simulink model "vegas01_4tap_1024ch_220_r8a"  (Simulink 7.6)
settings: Solver=ode45  StartTime=0.0  StopTime=128000  RelTol=1e-3
libraries: xbsIndex_r4 x13459, monroe_library x80, xps_library x51, casper_library_misc x43, xbsReplacements_r4 x42, dspsigops x16, simulink x7
(part 4 of 99)

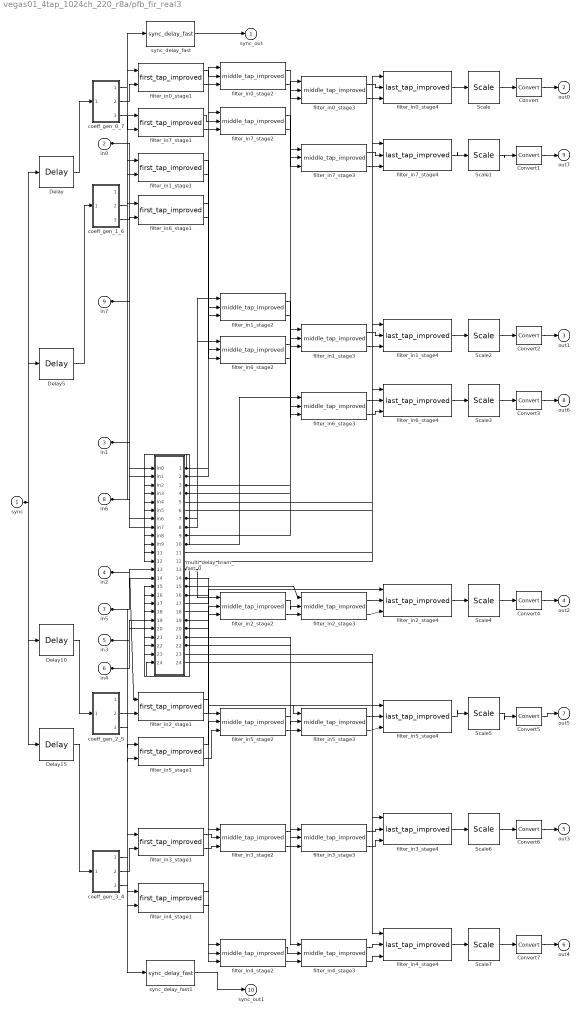
== system vegas01_4tap_1024ch_220_r8a/pfb_fir_real3 ==
  sync : Inport
  in0 : Inport  Port=2
  in1 : Inport  Port=3
  in2 : Inport  Port=4
  in3 : Inport  Port=5
  in4 : Inport  Port=6
  in5 : Inport  Port=7
  in6 : Inport  Port=8
  in7 : Inport  Port=9
  Convert{,1..7} (8 alike) : xbsIndex_r4/Convert  gui_display_data_type=Fixed-point  arith_type="Signed  (2's comp)"  n_bits=18  bin_pt=17  float_type=Single  exp_bits=8  fraction_bits=24  quantization=Truncate  overflow=Wrap  en=off  latency=0  dbl_ovrd=off  pipeline=off  block_type=convert
  Delay{,5,10,15} (4 alike) : xbsIndex_r4/Delay  rst=off  infoeditControl=Selection of Reset will increase slice count due to use of real FFs and instead of SRLs  en=off  latency=6  dbl_ovrd=off  reg_retiming=off  block_type=delay
  Scale{,1..7} (8 alike) : xbsIndex_r4/Scale  scale_factor=-1  dbl_ovrd=off  block_type=scale
  coeff_gen_0_7 : Subsystem
  coeff_gen_1_6 : Subsystem
  coeff_gen_2_5 : Subsystem
  coeff_gen_3_4 : Subsystem
  filter_in0_stage1 : monroe_library/first_tap_improved  SystemSampleTime=-1  data_bin_pt=7  coeff_bit_width=18
  filter_in0_stage2 : monroe_library/middle_tap_improved  SystemSampleTime=-1  data_bin_pt=7  coeff_bit_width=18  stage_num=2
  filter_in0_stage3 : monroe_library/middle_tap_improved  SystemSampleTime=-1  data_bin_pt=7  coeff_bit_width=18  stage_num=3
  filter_in0_stage4 : monroe_library/last_tap_improved  SystemSampleTime=-1  data_bin_pt=7  coeff_bin_pt=17  stage_num=4
  filter_in1_stage1 : monroe_library/first_tap_improved  SystemSampleTime=-1  data_bin_pt=7  coeff_bit_width=18
  filter_in1_stage2 : monroe_library/middle_tap_improved  SystemSampleTime=-1  data_bin_pt=7  coeff_bit_width=18  stage_num=2
  filter_in1_stage3 : monroe_library/middle_tap_improved  SystemSampleTime=-1  data_bin_pt=7  coeff_bit_width=18  stage_num=3
  filter_in1_stage4 : monroe_library/last_tap_improved  SystemSampleTime=-1  data_bin_pt=7  coeff_bin_pt=17  stage_num=4
  filter_in2_stage1 : monroe_library/first_tap_improved  SystemSampleTime=-1  data_bin_pt=7  coeff_bit_width=18
  filter_in2_stage2 : monroe_library/middle_tap_improved  SystemSampleTime=-1  data_bin_pt=7  coeff_bit_width=18  stage_num=2
  filter_in2_stage3 : monroe_library/middle_tap_improved  SystemSampleTime=-1  data_bin_pt=7  coeff_bit_width=18  stage_num=3
  filter_in2_stage4 : monroe_library/last_tap_improved  SystemSampleTime=-1  data_bin_pt=7  coeff_bin_pt=17  stage_num=4
  filter_in3_stage1 : monroe_library/first_tap_improved  SystemSampleTime=-1  data_bin_pt=7  coeff_bit_width=18
  filter_in3_stage2 : monroe_library/middle_tap_improved  SystemSampleTime=-1  data_bin_pt=7  coeff_bit_width=18  stage_num=2
  filter_in3_stage3 : monroe_library/middle_tap_improved  SystemSampleTime=-1  data_bin_pt=7  coeff_bit_width=18  stage_num=3
  filter_in3_stage4 : monroe_library/last_tap_improved  SystemSampleTime=-1  data_bin_pt=7  coeff_bin_pt=17  stage_num=4
  filter_in4_stage1 : monroe_library/first_tap_improved  SystemSampleTime=-1  data_bin_pt=7  coeff_bit_width=18
  filter_in4_stage2 : monroe_library/middle_tap_improved  SystemSampleTime=-1  data_bin_pt=7  coeff_bit_width=18  stage_num=2
  filter_in4_stage3 : monroe_library/middle_tap_improved  SystemSampleTime=-1  data_bin_pt=7  coeff_bit_width=18  stage_num=3
  filter_in4_stage4 : monroe_library/last_tap_improved  SystemSampleTime=-1  data_bin_pt=7  coeff_bin_pt=17  stage_num=4
  filter_in5_stage1 : monroe_library/first_tap_improved  SystemSampleTime=-1  data_bin_pt=7  coeff_bit_width=18
  filter_in5_stage2 : monroe_library/middle_tap_improved  SystemSampleTime=-1  data_bin_pt=7  coeff_bit_width=18  stage_num=2
  filter_in5_stage3 : monroe_library/middle_tap_improved  SystemSampleTime=-1  data_bin_pt=7  coeff_bit_width=18  stage_num=3
  filter_in5_stage4 : monroe_library/last_tap_improved  SystemSampleTime=-1  data_bin_pt=7  coeff_bin_pt=17  stage_num=4
  filter_in6_stage1 : monroe_library/first_tap_improved  SystemSampleTime=-1  data_bin_pt=7  coeff_bit_width=18
  filter_in6_stage2 : monroe_library/middle_tap_improved  SystemSampleTime=-1  data_bin_pt=7  coeff_bit_width=18  stage_num=2
  filter_in6_stage3 : monroe_library/middle_tap_improved  SystemSampleTime=-1  data_bin_pt=7  coeff_bit_width=18  stage_num=3
  filter_in6_stage4 : monroe_library/last_tap_improved  SystemSampleTime=-1  data_bin_pt=7  coeff_bin_pt=17  stage_num=4
  filter_in7_stage1 : monroe_library/first_tap_improved  SystemSampleTime=-1  data_bin_pt=7  coeff_bit_width=18
  filter_in7_stage2 : monroe_library/middle_tap_improved  SystemSampleTime=-1  data_bin_pt=7  coeff_bit_width=18  stage_num=2
  filter_in7_stage3 : monroe_library/middle_tap_improved  SystemSampleTime=-1  data_bin_pt=7  coeff_bit_width=18  stage_num=3
  filter_in7_stage4 : monroe_library/last_tap_improved  SystemSampleTime=-1  data_bin_pt=7  coeff_bin_pt=17  stage_num=4
  multi_delay_bram_fast_0 : Subsystem
  sync_delay_fast : monroe_library/sync_delay_fast  SystemSampleTime=-1  delay_len=768
      link override Constant2: opselect=C  inp2=PCIN>>17  inp1=P  carry=CIN
  sync_delay_fast1 : monroe_library/sync_delay_fast  SystemSampleTime=-1  delay_len=768
      link override Constant2: opselect=C  inp2=PCIN>>17  inp1=P  carry=CIN
  sync_out : Outport
  out0 : Outport  Port=2
  out1 : Outport  Port=3
  out2 : Outport  Port=4
  out3 : Outport  Port=5
  out4 : Outport  Port=6
  out5 : Outport  Port=7
  out6 : Outport  Port=8
  out7 : Outport  Port=9
  sync_out1 : Outport  Port=10
  wires:
  sync/1 -> Delay15/1, Delay10/1, Delay5/1, Delay/1
  in0/1 -> multi_delay_bram_fast_0/1(in0), filter_in0_stage1/1
  in7/1 -> multi_delay_bram_fast_0/2(in1), filter_in7_stage1/1
  in1/1 -> filter_in1_stage1/1, multi_delay_bram_fast_0/7(in6)
  in6/1 -> filter_in6_stage1/1, multi_delay_bram_fast_0/8(in7)
  in2/1 -> filter_in2_stage1/1, multi_delay_bram_fast_0/13(in12)
  in5/1 -> filter_in5_stage1/1, multi_delay_bram_fast_0/14(in13)
  in3/1 -> filter_in3_stage1/1, multi_delay_bram_fast_0/19(in18)
  in4/1 -> filter_in4_stage1/1, multi_delay_bram_fast_0/20(in19)
  multi_delay_bram_fast_0/1(out0) -> filter_in0_stage2/1, multi_delay_bram_fast_0/3(in2)
  multi_delay_bram_fast_0/2(out1) -> filter_in7_stage2/1, multi_delay_bram_fast_0/4(in3)
  multi_delay_bram_fast_0/3(out2) -> filter_in0_stage3/1, multi_delay_bram_fast_0/5(in4)
  multi_delay_bram_fast_0/4(out3) -> filter_in7_stage3/1, multi_delay_bram_fast_0/6(in5)
  multi_delay_bram_fast_0/7(out6) -> filter_in1_stage2/1, multi_delay_bram_fast_0/9(in8)
  multi_delay_bram_fast_0/8(out7) -> filter_in6_stage2/1, multi_delay_bram_fast_0/10(in9)
  multi_delay_bram_fast_0/9(out8) -> filter_in1_stage3/1, multi_delay_bram_fast_0/11(in10)
  multi_delay_bram_fast_0/10(out9) -> filter_in6_stage3/1, multi_delay_bram_fast_0/12(in11)
  multi_delay_bram_fast_0/13(out12) -> filter_in2_stage2/1, multi_delay_bram_fast_0/15(in14)
  multi_delay_bram_fast_0/14(out13) -> filter_in5_stage2/1, multi_delay_bram_fast_0/16(in15)
  multi_delay_bram_fast_0/15(out14) -> filter_in2_stage3/1, multi_delay_bram_fast_0/17(in16)
  multi_delay_bram_fast_0/16(out15) -> filter_in5_stage3/1, multi_delay_bram_fast_0/18(in17)
  multi_delay_bram_fast_0/19(out18) -> filter_in3_stage2/1, multi_delay_bram_fast_0/21(in20)
  multi_delay_bram_fast_0/20(out19) -> filter_in4_stage2/1, multi_delay_bram_fast_0/22(in21)
  multi_delay_bram_fast_0/21(out20) -> filter_in3_stage3/1, multi_delay_bram_fast_0/23(in22)
  multi_delay_bram_fast_0/22(out21) -> filter_in4_stage3/1, multi_delay_bram_fast_0/24(in23)
  sync_delay_fast1/1 -> sync_out1/1
  coeff_gen_3_4/1(sync_out) -> sync_delay_fast1/1
  sync_delay_fast/1 -> sync_out/1
  coeff_gen_0_7/1(sync_out) -> sync_delay_fast/1
  Convert7/1 -> out4/1
  Scale7/1 -> Convert7/1
  Convert6/1 -> out3/1
  Scale6/1 -> Convert6/1
  filter_in4_stage4/1 -> Scale7/1
  filter_in3_stage4/1 -> Scale6/1
  filter_in4_stage3/2 -> filter_in4_stage4/3
  filter_in4_stage3/1 -> filter_in4_stage4/2
  multi_delay_bram_fast_0/24(out23) -> filter_in4_stage4/1
  filter_in3_stage3/2 -> filter_in3_stage4/3
  filter_in3_stage3/1 -> filter_in3_stage4/2
  multi_delay_bram_fast_0/23(out22) -> filter_in3_stage4/1
  filter_in4_stage2/2 -> filter_in4_stage3/3
  filter_in4_stage2/1 -> filter_in4_stage3/2
  filter_in3_stage2/2 -> filter_in3_stage3/3
  filter_in3_stage2/1 -> filter_in3_stage3/2
  filter_in4_stage1/2 -> filter_in4_stage2/3
  filter_in4_stage1/1 -> filter_in4_stage2/2
  filter_in3_stage1/2 -> filter_in3_stage2/3
  filter_in3_stage1/1 -> filter_in3_stage2/2
  coeff_gen_3_4/3(coeff_rev) -> filter_in4_stage1/2
  coeff_gen_3_4/2(coeff) -> filter_in3_stage1/2
  Delay15/1 -> coeff_gen_3_4/1(sync)
  Convert5/1 -> out5/1
  Scale5/1 -> Convert5/1
  Convert4/1 -> out2/1
  Scale4/1 -> Convert4/1
  filter_in5_stage4/1 -> Scale5/1
  filter_in2_stage4/1 -> Scale4/1
  filter_in5_stage3/2 -> filter_in5_stage4/3
  filter_in5_stage3/1 -> filter_in5_stage4/2
  multi_delay_bram_fast_0/18(out17) -> filter_in5_stage4/1
  filter_in2_stage3/2 -> filter_in2_stage4/3
  filter_in2_stage3/1 -> filter_in2_stage4/2
  multi_delay_bram_fast_0/17(out16) -> filter_in2_stage4/1
  filter_in5_stage2/2 -> filter_in5_stage3/3
  filter_in5_stage2/1 -> filter_in5_stage3/2
  filter_in2_stage2/2 -> filter_in2_stage3/3
  filter_in2_stage2/1 -> filter_in2_stage3/2
  filter_in5_stage1/2 -> filter_in5_stage2/3
  filter_in5_stage1/1 -> filter_in5_stage2/2
  filter_in2_stage1/2 -> filter_in2_stage2/3
  filter_in2_stage1/1 -> filter_in2_stage2/2
  coeff_gen_2_5/3(coeff_rev) -> filter_in5_stage1/2
  coeff_gen_2_5/2(coeff) -> filter_in2_stage1/2
  Delay10/1 -> coeff_gen_2_5/1(sync)
  Convert3/1 -> out6/1
  Scale3/1 -> Convert3/1
  Convert2/1 -> out1/1
  Scale2/1 -> Convert2/1
  filter_in6_stage4/1 -> Scale3/1
  filter_in1_stage4/1 -> Scale2/1
  filter_in6_stage3/2 -> filter_in6_stage4/3
  filter_in6_stage3/1 -> filter_in6_stage4/2
  multi_delay_bram_fast_0/12(out11) -> filter_in6_stage4/1
  filter_in1_stage3/2 -> filter_in1_stage4/3
  filter_in1_stage3/1 -> filter_in1_stage4/2
  multi_delay_bram_fast_0/11(out10) -> filter_in1_stage4/1
  filter_in6_stage2/2 -> filter_in6_stage3/3
  filter_in6_stage2/1 -> filter_in6_stage3/2
  filter_in1_stage2/2 -> filter_in1_stage3/3
  filter_in1_stage2/1 -> filter_in1_stage3/2
  filter_in6_stage1/2 -> filter_in6_stage2/3
  filter_in6_stage1/1 -> filter_in6_stage2/2
  filter_in1_stage1/2 -> filter_in1_stage2/3
  filter_in1_stage1/1 -> filter_in1_stage2/2
  coeff_gen_1_6/3(coeff_rev) -> filter_in6_stage1/2
  coeff_gen_1_6/2(coeff) -> filter_in1_stage1/2
  Delay5/1 -> coeff_gen_1_6/1(sync)
  Convert1/1 -> out7/1
  Scale1/1 -> Convert1/1
  Convert/1 -> out0/1
  Scale/1 -> Convert/1
  filter_in7_stage4/1 -> Scale1/1
  filter_in0_stage4/1 -> Scale/1
  filter_in7_stage3/2 -> filter_in7_stage4/3
  filter_in7_stage3/1 -> filter_in7_stage4/2
  multi_delay_bram_fast_0/6(out5) -> filter_in7_stage4/1
  filter_in0_stage3/2 -> filter_in0_stage4/3
  filter_in0_stage3/1 -> filter_in0_stage4/2
  multi_delay_bram_fast_0/5(out4) -> filter_in0_stage4/1
  filter_in7_stage2/2 -> filter_in7_stage3/3
  filter_in7_stage2/1 -> filter_in7_stage3/2
  filter_in0_stage2/2 -> filter_in0_stage3/3
  filter_in0_stage2/1 -> filter_in0_stage3/2
  filter_in7_stage1/2 -> filter_in7_stage2/3
  filter_in7_stage1/1 -> filter_in7_stage2/2
  filter_in0_stage1/2 -> filter_in0_stage2/3
  filter_in0_stage1/1 -> filter_in0_stage2/2
  coeff_gen_0_7/3(coeff_rev) -> filter_in7_stage1/2
  coeff_gen_0_7/2(coeff) -> filter_in0_stage1/2
  Delay/1 -> coeff_gen_0_7/1(sync)

== system vegas01_4tap_1024ch_220_r8a/pktsnap ==
  din : Inport
  trig : Inport  Port=2
  we : Inport  Port=3
  Constant : xbsIndex_r4/Constant  const=1  gui_display_data_type=Fixed-point  arith_type=Boolean  n_bits=1  bin_pt=0  preci_type=Single  exp_width=8  frac_width=24  explicit_period=on  period=1  equ=P=C  opselect=C  inp2=PCIN>>17  opr=+  inp1=P  carry=CIN  dbl_ovrd=off  block_type=constant
      settings: block_version=10.1.1
  Constant1 : xbsIndex_r4/Constant  const=1  gui_display_data_type=Fixed-point  arith_type=Boolean  n_bits=1  bin_pt=0  preci_type=Single  exp_width=8  frac_width=24  explicit_period=on  period=1  equ=P=C  opselect=C  inp2=PCIN>>17  opr=+  inp1=P  carry=CIN  dbl_ovrd=off  block_type=constant
      settings: block_version=10.1.1
  Constant13 : Constant
  Constant2 : xbsIndex_r4/Constant  const=1  gui_display_data_type=Fixed-point  arith_type=Boolean  n_bits=1  bin_pt=0  preci_type=Single  exp_width=8  frac_width=24  explicit_period=on  period=1  equ=P=C  opselect=C  inp2=PCIN>>17  opr=+  inp1=P  carry=CIN  dbl_ovrd=off  block_type=constant
      settings: block_version=10.1.1
  Inverter : xbsIndex_r4/Inverter  en=off  latency=0  dbl_ovrd=off  block_type=inv
      settings: xl_area=[0, 0, 0, 0, 0, 0, 0]  block_version=10.1.1
  Logical1 : xbsIndex_r4/Logical  logical_function=AND  inputs=2  en=off  latency=0  precision=Full  arith_type=Unsigned  n_bits=8  bin_pt=2  align_bp=on  dbl_ovrd=off  block_type=logical
      settings: xl_area=[0, 0, 0, 0, 0, 0, 0]  block_version=10.1.1
  Mux1 : xbsIndex_r4/Mux  inputs=2  en=off  latency=0  precision=Full  arith_type="Signed  (2's comp)"  n_bits=8  bin_pt=2  quantization=Truncate  overflow=Wrap  dbl_ovrd=off  block_type=mux
      settings: xl_area=[0, 0, 0, 0, 0, 0, 0]  block_version=10.1.1
  Mux2 : xbsIndex_r4/Mux  inputs=2  en=off  latency=0  precision=Full  arith_type="Signed  (2's comp)"  n_bits=8  bin_pt=2  quantization=Truncate  overflow=Wrap  dbl_ovrd=off  block_type=mux
      settings: xl_area=[0, 0, 0, 0, 0, 0, 0]  block_version=10.1.1
  Register : xbsIndex_r4/Register  init=0  rst=on  en=on  dbl_ovrd=off  block_type=register
      settings: xl_area=[0, 0, 0, 0, 0, 0, 0]  block_version=10.1.1
  Register1 : xbsIndex_r4/Register  init=0  rst=on  en=on  dbl_ovrd=off  block_type=register
      settings: xl_area=[0, 0, 0, 0, 0, 0, 0]  block_version=10.1.1
  Reinterpret3 : xbsIndex_r4/Reinterpret  force_arith_type=on  arith_type=Unsigned  force_bin_pt=on  bin_pt=0  block_type=reinterpret
      settings: infoedit="Changes signal type without altering the binary representation.   You can changed the signal between signed and unsigned, and relocate the binary point.<P><P>Hardware notes: In hardware this block costs nothing.<P><P>Example:  Suppose the input is 6 bits wide, signed, with 2 fractional bits, and the output is forced to unsigned with 0 fractional bits.  Then an input of -2.0 (1110.00 in binary 2's complement) becomes an output of 56 (111000 in binary)."  block_version=10.1.1
  addr : xps_library/software register  Tag=xps:sw_reg  SystemSampleTime=-1  io_dir=To Processor  arith_type=Unsigned  bitwidth=32  bin_pt=0  sample_period=1  gw_name=vegas01_4tap_1024ch_220_r8a_pktsnap_addr_user_data_in
  bram_lsb : Subsystem  Tag=xps:bram  AncestorBlock=xps_library/Shared BRAM  mask{arith_type=Unsigned, addr_width=nsamples, data_width=32, data_bin_pt=0, init_vals=0, sample_rate=1}
      mask prompts: arith_type "Output Data Type"; addr_width "Address width"; data_width "Data Width"; data_bin_pt "Data Binary Point"; init_vals "Initial 32-bit values (simulation only)"; sample_rate "Sample rate"
      mask init:
      | shared_bram_mask;
      mask StyleString: popup(Unsigned|Signed  (2's comp)),edit,popup(8|16|32|64|128),edit,edit,edit
  bram_msb : Subsystem  Tag=xps:bram  AncestorBlock=xps_library/Shared BRAM  mask{arith_type=Unsigned, addr_width=nsamples, data_width=32, data_bin_pt=0, init_vals=0, sample_rate=1}
      mask prompts: arith_type "Output Data Type"; addr_width "Address width"; data_width "Data Width"; data_bin_pt "Data Binary Point"; init_vals "Initial 32-bit values (simulation only)"; sample_rate "Sample rate"
      mask init:
      | shared_bram_mask;
      mask StyleString: popup(Unsigned|Signed  (2's comp)),edit,popup(8|16|32|64|128),edit,edit,edit
  ctrl : xps_library/software register  Tag=xps:sw_reg  SystemSampleTime=-1  io_dir=From Processor  arith_type=Unsigned  bitwidth=32  bin_pt=0  sample_period=1  gw_name=vegas01_4tap_1024ch_220_r8a_pktsnap_ctrl_user_data_out
  en1 : xbsIndex_r4/Slice  nbits=32  boolean_output=off  mode=Lower Bit Location + Width  bit1=0  base1=LSB of Input  bit0=32  base0=LSB of Input  dbl_ovrd=off  block_type=slice
      settings: infoedit="Extracts a given range of bits from each input sample and presents it at the output.  The output type is ordinarily unsigned with binary point at zero, but can be Boolean when the slice is one bit wide.<P><P>Hardware notes: In hardware this block costs nothing."  block_version=10.1.1
  en2 : xbsIndex_r4/Slice  nbits=32  boolean_output=off  mode=Lower Bit Location + Width  bit1=0  base1=LSB of Input  bit0=0  base0=LSB of Input  dbl_ovrd=off  block_type=slice
      settings: infoedit="Extracts a given range of bits from each input sample and presents it at the output.  The output type is ordinarily unsigned with binary point at zero, but can be Boolean when the slice is one bit wide.<P><P>Hardware notes: In hardware this block costs nothing."  block_version=10.1.1
  enable : xbsIndex_r4/Slice  nbits=1  boolean_output=on  mode=Lower Bit Location + Width  bit1=0  base1=LSB of Input  bit0=0  base0=LSB of Input  dbl_ovrd=off  block_type=slice
      settings: infoedit="Extracts a given range of bits from each input sample and presents it at the output.  The output type is ordinarily unsigned with binary point at zero, but can be Boolean when the slice is one bit wide.<P><P>Hardware notes: In hardware this block costs nothing."  block_version=10.1.1
  freeze_cntr : casper_library_misc/freeze_cntr  SystemSampleTime=-1  CounterBits=nsamples
  posedge : casper_library_misc/posedge  Tag=Rising Edge Detector  SystemSampleTime=-1
  trig1 : xbsIndex_r4/Slice  nbits=1  boolean_output=on  mode=Lower Bit Location + Width  bit1=0  base1=LSB of Input  bit0=1  base0=LSB of Input  dbl_ovrd=off  block_type=slice
      settings: infoedit="Extracts a given range of bits from each input sample and presents it at the output.  The output type is ordinarily unsigned with binary point at zero, but can be Boolean when the slice is one bit wide.<P><P>Hardware notes: In hardware this block costs nothing."  block_version=10.1.1
  valid : xbsIndex_r4/Slice  nbits=1  boolean_output=on  mode=Lower Bit Location + Width  bit1=0  base1=LSB of Input  bit0=2  base0=LSB of Input  dbl_ovrd=off  block_type=slice
      settings: infoedit="Extracts a given range of bits from each input sample and presents it at the output.  The output type is ordinarily unsigned with binary point at zero, but can be Boolean when the slice is one bit wide.<P><P>Hardware notes: In hardware this block costs nothing."  block_version=10.1.1
  wires:
  en2/1 -> bram_lsb/2(data_in)
  en1/1 -> bram_msb/2(data_in)
  din/1 -> Reinterpret3/1
  Reinterpret3/1 -> en2/1, en1/1
  trig/1 -> Mux2/2
  enable/1 -> posedge/1
  freeze_cntr/2 -> Register1/3, bram_msb/3(we), bram_lsb/3(we)
  Constant13/1 -> ctrl/1
  ctrl/1 -> enable/1, valid/1, trig1/1
  posedge/1 -> Register/3, Register1/2, Inverter/1
  Constant/1 -> Register/1
  Constant1/1 -> Mux2/3
  Mux1/1 -> freeze_cntr/1
  we/1 -> Mux1/2
  Constant2/1 -> Mux1/3
  Register1/1 -> addr/1
  trig1/1 -> Mux2/1
  valid/1 -> Mux1/1
  Logical1/1 -> Register/2
  Inverter/1 -> Logical1/2
  Mux2/1 -> Logical1/1
  Register/1 -> freeze_cntr/2
  freeze_cntr/1 -> Register1/1, bram_msb/1(addr), bram_lsb/1(addr)

== system vegas01_4tap_1024ch_220_r8a/reshaper ==
  note: reset will be tied to negative edge
      | of vacc
  note: narrower data width must go to port A of dual port ram
  rst : Inport
  valid : Inport  Port=2
  din : Inport  Port=3
  Constant : xbsIndex_r4/Constant  const=0  gui_display_data_type=Fixed-point  arith_type=Unsigned  n_bits=outwid  bin_pt=0  preci_type=Single  exp_width=8  frac_width=24  explicit_period=on  period=1  equ=P=C  opselect=C  inp2=PCIN>>17  opr=+  inp1=P  carry=CIN  dbl_ovrd=off  block_type=constant
      settings: block_version=11.4
  Constant2 : xbsIndex_r4/Constant  const=0  gui_display_data_type=Fixed-point  arith_type=Boolean  n_bits=outwid  bin_pt=0  preci_type=Single  exp_width=8  frac_width=24  explicit_period=on  period=1  equ=P=C  opselect=C  inp2=PCIN>>17  opr=+  inp1=P  carry=CIN  dbl_ovrd=off  block_type=constant
      settings: block_version=11.4
  Constant3 : xbsIndex_r4/Constant  const=headlen  gui_display_data_type=Fixed-point  arith_type=Unsigned  n_bits=l2headlen  bin_pt=0  preci_type=Single  exp_width=8  frac_width=24  explicit_period=on  period=1  equ=P=C  opselect=C  inp2=PCIN>>17  opr=+  inp1=P  carry=CIN  dbl_ovrd=off  block_type=constant
      settings: block_version=11.4
  Counter : xbsIndex_r4/Counter  cnt_type=Free Running  cnt_to=Inf  operation=Up  start_count=0  cnt_by_val=1  arith_type=Unsigned  n_bits=l2inlen  bin_pt=0  load_pin=off  rst=on  en=on  explicit_period=on  period=1  dbl_ovrd=off  use_behavioral_HDL=off  implementation=Fabric  block_type=counter
      settings: block_version=11.4
  Counter3 : xbsIndex_r4/Counter  cnt_type=Free Running  cnt_to=1023  operation=Up  start_count=0  cnt_by_val=1  arith_type=Unsigned  n_bits=l2headlen + 1  bin_pt=0  load_pin=off  rst=on  en=on  explicit_period=on  period=1  dbl_ovrd=off  use_behavioral_HDL=off  implementation=Fabric  use_rpm=on  block_type=counter
      settings: xl_area=[0, 0, 0, 0, 0, 0, 0]  block_version=9.1.01
  Delay : xbsIndex_r4/Delay  rst=off  infoeditControl=Selection of Reset will increase slice count due to use of real FFs and instead of SRLs  en=off  latency=1  dbl_ovrd=off  reg_retiming=off  block_type=delay
      settings: block_version=11.4
  Delay1 : xbsIndex_r4/Delay  rst=off  infoeditControl=Selection of Reset will increase slice count due to use of real FFs and instead of SRLs  en=off  latency=2  dbl_ovrd=off  reg_retiming=off  block_type=delay
      settings: block_version=11.4
  Delay2 : xbsIndex_r4/Delay  rst=off  infoeditControl=Selection of Reset will increase slice count due to use of real FFs and instead of SRLs  en=off  latency=2  dbl_ovrd=off  reg_retiming=off  block_type=delay
      settings: block_version=11.4
  Dual Port RAM : xbsIndex_r4/Dual Port RAM  depth=outlen  initVector=sin(pi*(0:outlen)/16)  distributed_mem=Block RAM  init_a=0  init_b=0  rst_a=off  rst_b=off  en_a=off  en_b=off  latency=2  write_mode_A=Read After Write  write_mode_B=Read After Write  dbl_ovrd=off  optimize=Speed  use_rpm=on  block_type=dpram
      settings: block_version=11.4
  Inverter : xbsIndex_r4/Inverter  en=off  latency=0  dbl_ovrd=off  block_type=inv
      settings: block_version=11.4
  Inverter1 : xbsIndex_r4/Inverter  en=off  latency=0  dbl_ovrd=off  block_type=inv
      settings: block_version=11.4
  Logical : xbsIndex_r4/Logical  logical_function=AND  inputs=2  en=off  latency=0  precision=Full  arith_type=Unsigned  n_bits=16  bin_pt=0  align_bp=on  dbl_ovrd=off  block_type=logical
      settings: block_version=11.4
  Logical1 : xbsIndex_r4/Logical  logical_function=AND  inputs=2  en=off  latency=0  precision=Full  arith_type=Unsigned  n_bits=16  bin_pt=0  align_bp=on  dbl_ovrd=off  block_type=logical
      settings: block_version=11.4
  MCode : xbsIndex_r4/MCode  mfname=reshaper_fsm.m  explicit_period=off  period=1  inputsTable={'boundInpExpr'=>['','','','',''],'inputs'=>['end_chunk','end_block','rst','valid_in','head_done']}  outputsTable={'outputs'=>['block_count_rst','chunk_count_rst','chunk_count_en','sel_rst','sel_en','header_valid','data_valid','st'],'suppressOut'=>['off','off','off','off','off','off','off','off']}  dbl_ovrd=off  enable_stdout=off  enable_debug=off  suppress_output=on  defparams={}  hide_port_list={}  block_type=mcode
      settings: block_version=11.4
  Relational : xbsIndex_r4/Relational  mode=a>=b  en=off  latency=0  dbl_ovrd=off  block_type=relational
  Terminator : Terminator
  block_counter : casper_library_misc/freeze_cntr  SystemSampleTime=-1  CounterBits=l2outlen
  block_done : xbsIndex_r4/Gateway Out  hdl_port=off  timing_constraint=None  locs_specified=off  LOCs={}  block_type=gatewayout
      settings: infoedit="Gateway out block.  Converts Xilinx fixed point inputs into ouputs of type Simulink integer, double, or fixed point.<br><br>Hardware notes:  In hardware these blocks become top level output ports or are discarded, depending on how they are configured."  block_version=11.4
  chunk_done_p : xbsIndex_r4/Gateway Out  hdl_port=off  timing_constraint=None  locs_specified=off  LOCs={}  block_type=gatewayout
      settings: infoedit="Gateway out block.  Converts Xilinx fixed point inputs into ouputs of type Simulink integer, double, or fixed point.<br><br>Hardware notes:  In hardware these blocks become top level output ports or are discarded, depending on how they are configured."  block_version=11.4
  chunkcounter : casper_library_misc/freeze_cntr  SystemSampleTime=-1  CounterBits=l2outchunk
  enable : xbsIndex_r4/Slice  nbits=l2headlen  boolean_output=off  mode=Lower Bit Location + Width  bit1=0  base1=LSB of Input  bit0=0  base0=LSB of Input  dbl_ovrd=off  block_type=slice
      settings: block_version=9.1.01
  head_done_p1 : xbsIndex_r4/Gateway Out  hdl_port=off  timing_constraint=None  locs_specified=off  LOCs={}  block_type=gatewayout
      settings: infoedit="Gateway out block.  Converts Xilinx fixed point inputs into ouputs of type Simulink integer, double, or fixed point.<br><br>Hardware notes:  In hardware these blocks become top level output ports or are discarded, depending on how they are configured."  block_version=11.4
  dout : Outport
  sel : Outport  Port=2
  header_valid_out : Outport  Port=3
  data_valid_out : Outport  Port=4
  st : Outport  Port=5
  wires:
  Logical1/1 -> Delay2/1
  MCode/7 -> Logical1/2
  Delay2/1 -> data_valid_out/1
  MCode/6 -> Delay1/1
  MCode/8 -> st/1
  [chunk_done] -> Inverter1/1
  Inverter1/1 -> Logical1/1
  [chunk_done] -> Inverter/1
  Inverter/1 -> Logical/1
  Logical/1 -> block_counter/1
  [blk_cnt_en] -> Logical/2
  Delay1/1 -> header_valid_out/1
  Delay/1 -> sel/1
  din/1 -> Dual Port RAM/5
  Counter/1 -> Dual Port RAM/4  "dinadddr"
  Constant2/1 -> Dual Port RAM/3
  Constant/1 -> Dual Port RAM/2
  block_counter/1 -> Dual Port RAM/1  "doutaddr"
  Dual Port RAM/2 -> Terminator/1
  Dual Port RAM/1 -> dout/1
  [valid] -> MCode/4
  [rst] -> MCode/3
  [head_done] -> MCode/5
  [block_done] -> MCode/2  "blockdone"
  [chunk_done] -> MCode/1  "chunkdone"
  MCode/4 -> [sel_cnt_rst]  "sel_cnt_rst"
  MCode/5 -> [sel_cnt_en]  "sel_cnt_en"
  Relational/1 -> head_done_p1/1, [head_done]  "headdone"
  enable/1 -> Delay/1
  [sel_cnt_en] -> Counter3/2
  Constant3/1 -> Relational/2
  Counter3/1 -> Relational/1, enable/1
  [sel_cnt_rst] -> Counter3/1
  MCode/2 -> [chunk_cnt_rst]  "chunk_cnt_rst"
  MCode/1 -> [blk_cnt_rst]  "blk_cnt_rst"
  MCode/3 -> [blk_cnt_en]  "chunk_count_nen"
  [blk_cnt_rst] -> block_counter/2  "blk_cnt_rst"
  block_counter/3 -> block_done/1, [block_done]  "block_dneone"
  [chunk_cnt_rst] -> chunkcounter/2  "chunk_cnt_rst"
  chunkcounter/3 -> chunk_done_p/1, [chunk_done]  "chunk_done"
  [blk_cnt_en] -> chunkcounter/1  "blk_cnt_en"
  valid/1 -> Dual Port RAM/6, [valid], Counter/2
  rst/1 -> [rst], Counter/1

== system vegas01_4tap_1024ch_220_r8a/sg ==
  note: sync_disable
  note: syn_sel
  note: soft_pps
  pps : Inport
  Constant : Constant
  Constant2 : Constant  Value=0
  Counter3 : xbsIndex_r4/Counter  cnt_type=Free Running  cnt_to=Inf  operation=Up  start_count=0  cnt_by_val=1  arith_type=Unsigned  n_bits=32  bin_pt=0  load_pin=off  rst=on  en=off  explicit_period=off  period=1  dbl_ovrd=off  use_behavioral_HDL=off  implementation=Fabric  use_rpm=on  block_type=counter
      settings: xl_area=[0, 0, 0, 0, 0, 0, 0]
  Inverter : xbsIndex_r4/Inverter  en=off  latency=0  dbl_ovrd=off  block_type=inv
      settings: block_version=11.4
  Logical1 : xbsIndex_r4/Logical  logical_function=OR  inputs=2  en=off  latency=0  precision=Full  arith_type=Unsigned  n_bits=8  bin_pt=2  align_bp=on  dbl_ovrd=off  block_type=logical
      settings: xl_area=[0, 0, 0, 0, 0, 0, 0]
  Logical2 : xbsIndex_r4/Logical  logical_function=OR  inputs=2  en=off  latency=1  precision=Full  arith_type=Unsigned  n_bits=8  bin_pt=2  align_bp=on  dbl_ovrd=off  block_type=logical
      settings: xl_area=[0, 0, 0, 0, 0, 0, 0]
  Logical3 : xbsIndex_r4/Logical  logical_function=AND  inputs=2  en=off  latency=0  precision=Full  arith_type=Unsigned  n_bits=8  bin_pt=2  align_bp=on  dbl_ovrd=off  block_type=logical
      settings: xl_area=[0, 0, 0, 0, 0, 0, 0]
  Logical4 : xbsIndex_r4/Logical  logical_function=AND  inputs=2  en=off  latency=0  precision=Full  arith_type=Unsigned  n_bits=8  bin_pt=2  align_bp=on  dbl_ovrd=off  block_type=logical
      settings: xl_area=[0, 0, 0, 0, 0, 0, 0]
  Mux : xbsIndex_r4/Mux  inputs=2  en=off  latency=1  precision=Full  arith_type=Unsigned  n_bits=16  bin_pt=14  quantization=Truncate  overflow=Wrap  dbl_ovrd=off  block_type=mux
  Register : xbsIndex_r4/Register  init=0  rst=off  en=off  dbl_ovrd=off  block_type=register
      settings: block_version=11.4
  Register1 : xbsIndex_r4/Register  init=0  rst=off  en=off  dbl_ovrd=off  block_type=register
      settings: block_version=11.4
  Register2 : xbsIndex_r4/Register  init=0  rst=off  en=off  dbl_ovrd=off  block_type=register
      settings: block_version=11.4
  Relational : xbsIndex_r4/Relational  mode=a<b  en=off  latency=1  dbl_ovrd=off  block_type=relational
      settings: xl_area=[0, 0, 0, 0, 0, 0, 0]  block_version=10.1.3
  bus_expand : Subsystem  AncestorBlock=casper_library_flow_control/bus_expand  mask(bus_expand){mode=divisions of equal size, outputNum=4, outputWidth=8, outputBinaryPt=0, outputArithmeticType=0, outputToWorkspace=off, variablePrefix=out, outputToModelAsWell=on}
      mask prompts: outputNum "Number of outputs:"; outputWidth "Output width:"; outputBinaryPt "Output binary point position:"; outputArithmeticType "Output arithmetic type: (Unsigned=0, Signed=1, Boolean=2)"; outputToWorkspace "Output to workspace?"; variablePrefix "Variable name prefix:"; outputToModelAsWell "Output to model as well?"
      mask help:
      | Expands a 'bus' made using a bus_create or Xilinx
      | concat block.
      | The input will be divided into the specified number
      | of outputs with the specified arithmetic types.
      | Divisions are made from most significant bit.
      mask init: same as vegas01_4tap_1024ch_220_r8a/bus_expand3
      settings: AttributesFormatString=4 outputs
      mask StyleString: popup(divisions of equal size|divisions of arbitrary size),edit,edit,edit,edit,checkbox,edit,checkbox
      mask CallbackString: bus_expand_callback();||bus_expand_callback();|||bus_expand_callback();||
  bus_expand1 : Subsystem  AncestorBlock=casper_library_flow_control/bus_expand  mask(bus_expand){mode=divisions of equal size, outputNum=8, outputWidth=1, outputBinaryPt=0, outputArithmeticType=2, outputToWorkspace=off, variablePrefix=out, outputToModelAsWell=on}
      mask prompts: outputNum "Number of outputs:"; outputWidth "Output width:"; outputBinaryPt "Output binary point position:"; outputArithmeticType "Output arithmetic type: (Unsigned=0, Signed=1, Boolean=2)"; outputToWorkspace "Output to workspace?"; variablePrefix "Variable name prefix:"; outputToModelAsWell "Output to model as well?"
      mask help:
      | Expands a 'bus' made using a bus_create or Xilinx
      | concat block.
      | The input will be divided into the specified number
      | of outputs with the specified arithmetic types.
      | Divisions are made from most significant bit.
      mask init: same as vegas01_4tap_1024ch_220_r8a/bus_expand3
      settings: AttributesFormatString=8 outputs
      mask StyleString: popup(divisions of equal size|divisions of arbitrary size),edit,edit,edit,edit,checkbox,edit,checkbox
      mask CallbackString: bus_expand_callback();||bus_expand_callback();|||bus_expand_callback();||
  delay1 : xbsIndex_r4/Register  init=0  rst=off  en=off  dbl_ovrd=off  block_type=register
      settings: block_version=11.4
  delay2 : xbsIndex_r4/Register  init=0  rst=off  en=off  dbl_ovrd=off  block_type=register
      settings: block_version=11.4
  period : xps_library/software register  Tag=xps:sw_reg  SystemSampleTime=-1  io_dir=From Processor  arith_type=Unsigned  bitwidth=32  bin_pt=0  sample_period=1  gw_name=vegas01_4tap_1024ch_220_r8a_sg_period_user_data_out
  posedge1 : casper_library_misc/posedge  Tag=Rising Edge Detector  SystemSampleTime=-1
  posedge3 : casper_library_misc/posedge  Tag=Rising Edge Detector  SystemSampleTime=-1
  sync : xps_library/software register  Tag=xps:sw_reg  SystemSampleTime=-1  io_dir=From Processor  arith_type=Unsigned  bitwidth=32  bin_pt=0  sample_period=1  gw_name=vegas01_4tap_1024ch_220_r8a_sg_sync_user_data_out
  sync_period_const : xbsIndex_r4/Constant  const=2046  gui_display_data_type=Fixed-point  arith_type=Unsigned  n_bits=32  bin_pt=0  preci_type=Single  exp_width=8  frac_width=24  explicit_period=off  period=1  equ=P=C  opselect=C  inp2=PCIN>>17  opr=+  inp1=P  carry=CIN  dbl_ovrd=off  block_type=constant
      settings: block_version=10.1.1
  sync_out : Outport
  pps_out : Outport  Port=2
  wires:
  Constant2/1 -> period/1
  period/1 -> Register2/1
  sync_period_const/1 -> Mux/2
  Mux/1 -> Relational/1
  Relational/1 -> Logical1/1  "syncrst"
  Counter3/1 -> Relational/2  "sync_count"
  Logical1/1 -> Counter3/1, Logical3/1
  Constant/1 -> sync/1
  posedge1/1 -> Register1/1
  sync/1 -> Register/1
  Register/1 -> bus_expand/1(bus_in)
  bus_expand/4(lsb_out1) -> bus_expand1/1(bus_in)
  bus_expand1/4(out5) -> Mux/1
  bus_expand1/8(lsb_out1) -> posedge3/1
  Logical2/1 -> delay1/1, delay2/1
  bus_expand1/7(out2) -> Inverter/1
  Logical3/1 -> posedge1/1
  Register1/1 -> sync_out/1
  Register2/1 -> Mux/3
  Inverter/1 -> Logical3/2
  pps/1 -> Logical4/2
  Logical4/1 -> Logical2/2
  bus_expand1/6(out3) -> Logical4/1
  posedge3/1 -> Logical2/1
  delay1/1 -> Logical1/2
  delay2/1 -> pps_out/1
